simulink model "HDVSim_Hybrid_Truck"  (R2019b)
settings: Solver=ode3  StartTime=0.0  StopTime=inf  FixedStep=sample_time  MaxStep=0.1  RelTol=1e-2  EnableMultiTasking=on
model workspace (MAT data in the model file):
  Bat.SOC_start = 1
  Bat.Voltage = 800
  Bat.Useable_range = 0.8
  Bat.Charge_cycles = 3000
  Bat.Useable_capacity = 61.538
  Bat.Efficiency_power_electronics = 0.95
  Bat.Type = 2
  Electric_Truck = 0
  FuelCell.P_nom = 0
  Fueltype = 4
  Hybrid_Truck = 1
  IZ12 = 3600
  M_el = 500
  SOC_T_EM_completely_available = 0.5
  SOC_addition = 0.4
  SOC_el = 0.8
  SOC_target = 0.85
  Sailing_without_drag_torque = 0
  TCO = TCO
  TCO.Ratio_Trailer_Truck = [1 1]
  TCO.Price_increase_repurchase = [3.5 3.5]
  TCO.bDiesel = 20.3670861225
  TCO.CNG_consumption = 0
  TCO.LNG_consumption = 0
  TCO.energyTotal = 12.8064463441
  TCO.Hydrogen_consumption = 0
  TCO.Daily_working_time = 8
  TCO.Other_works_day = 0.5
  TCO.Gross_hourly_wage_driver = 13
  TCO.Operating_days_year = 240
  TCO.Annual_gross_wage_driver = 24960
  TCO.Personnel_factor = 1.3
  TCO.Annual_gross_wage_truck = 32448
  TCO.Social_costs_percent = 25.75
  TCO.Social_costs_EUR = 8355
  TCO.Back_up_driver = 0
  TCO.Fees_day = 12
  TCO.Fees_year = 2880
  TCO.Driver_costs_other = 0
  TCO.Driving_personnel_costs_total = 43683
  TCO.Diesel_price = 0.95
  TCO.Diesel_price_external = 1
  TCO.Adblue_price = 0.68
  TCO.CNG_price = 0.88
  TCO.LNG_price = 0.81
  TCO.Electricity_price = 0.12
  TCO.Hydrogen_price = 9
  TCO.Interest_current_asset = 8.4
  TCO.Interest_fixed_asset = 8.4
  TCO.Administrative_costs = 10.7
  TCO.Driving_distance = 0
  TCO.Ratio_toll_route = 75
  TCO.Loading_time_combination = 0.25
  TCO.Unloading_time_combination = 0.25
  TCO.Ratio_empty_trips = 0
  TCO.Operating_life = [6 12]
  TCO.Tire_mileage = [140000 170000]
  TCO.Ratio_inhouse_tanking_diesel = 75
  TCO.Lubricant_consumption = 1
  TCO.Adblue_consumption = 4
  TCO.Afa_time_dependent = [100 100]
  TCO.Afa_power_dependent = [0 0]
  TCO.Current_asset = [6000 1400]
  TCO.Insurance_vehicle = [0 0]
  TCO.Insurance_transport = 1000
  TCO.Communication = 600
  TCO.Repair_maintenance_care = [0.13 0.025]
  TCO.Current_assets_interest_bearing = 622
  TCO.Results = table
  TCO.Results.data = {{[]; []; []; []; []; []; []; []; []; []; []; []; []; []; []; []; []; []; []; []; []; []; []}, {[]; []; []; []; []; []; []; []; []; []; []; []; []; []; []; []; []; []; []; []; []; []; []}, {[]; []; []; []; []; []; []; []; []; []; []; []; []; []; []; []; []; []; []; []; []; []; []}, {'#cccccc'; '#9DFF00'; '#CEFF00'; '#FFFF00'; '#FFDF00'; '#FFB900'; '#FF9200'; '#FF5D00'; '#FF7F7F'; '#FF2900'; '#cccccc'; '#00FF85'; '#00FFC6'; '#00FFF2'; '#00FFFF'; '#00DBFF'; '#00B3FF'; '#0088FF'; '#cccccc'; '#B100FF'; '#FF00FF'; '#FF00C0'; '#FF0070'}, [0; 1; 1; 1; 1; 1; 1; 0; 1; 0; 0; 1; 1; 1; 1; 1; 1; 0; 0; 0; 1; 1; 0]}
  TCO.Results.ndims = 2
  TCO.Results.nrows = 23
  TCO.Results.rownames = {'Time_dependent_vehicle_costs'; 'Amortisation_time_dependent'; 'Current_assets_interest_bearing'; 'Fixed_assets_interest_bearing'; 'Taxes'; 'Insurance'; 'Communication'; 'Sum_vehicle_costs'; 'Driving_personnel_costs_total'; 'Sum_fix_costs'; 'Performance_related_vehicle_costs'; 'Amortisation_performance_related'; 'Fuel_costs'; 'Adblue_costs'; 'Repair_costs'; 'Lubricant_costs'; 'Tire_costs'; 'Sum_variable_costs'; 'Sum_costs'; 'Sum_fix_variable_costs'; 'Overhead_costs'; 'Toll_costs'; 'Total_costs'}
  TCO.Results.nvars = 5
  TCO.Results.varnames = {'Euro_Year', 'Euro_km', 'Percent', 'Cellcolor', 'Diagramm'}
  TCO.Results.props.useVariableNamesOriginal = 0
  TCO.Results.props.useDimensionNamesOriginal = 0
  TCO.Results.props.CustomProps = struct()
  TCO.Results.props.VariableCustomProps = struct()
  TCO.Results.props.versionSavedFrom = 4
  TCO.Results.props.minCompatibleVersion = 1
  TCO.Results.props.incompatibilityMsg = ''
  TCO.Results.props.VersionSavedFrom = 4
  TCO.Results.props.Description = ''
  TCO.Results.props.VariableNamesOriginal = {}
  TCO.Results.props.DimensionNames = {'Row', 'Variables'}
  TCO.Results.props.DimensionNamesOriginal = {}
  TCO.Results.props.UserData = []
  TCO.Results.props.VariableDescriptions = {}
  TCO.Results.props.VariableUnits = {}
  TCO.Results.props.VariableContinuity = {}
  TCO_Trailer = 1
  T_distance_LPS_down = 250
  T_distance_LPS_up = 250
  VSim.Display = 3
  VSim.Opt = 0
  VSim.Termination = 1
  Vehicle = 'empty'
  WPT.Voltage = 0
  WPT.P_max = 120
  WPT.expansion = 0.42
  WPT.eta = 0.92
  WPT.SOC_target = 1
  WPT.SOC_electric_only = 1
  acquisitionCosts = acquisitionCosts
  acquisitionCosts.KA_ZM = 128696.075491
  acquisitionCosts.KG = 6766.52227329
  acquisitionCosts.Getr_m = 306.461675078
  acquisitionCosts.KMot = 12284.8
  acquisitionCosts.Motor_Mmax = 2400
  acquisitionCosts.Motor_m = 1122.29
  acquisitionCosts.KH = 20987.6378271
  acquisitionCosts.Hybrid = 1
  acquisitionCosts.Bat = 15579.57696
  acquisitionCosts.Bat_type = 2
  acquisitionCosts.Bat_m = 382.223602484
  acquisitionCosts.Bat_kWh = 61.538
  acquisitionCosts.Bat_VK_Pouch = 176
  acquisitionCosts.Bat_VK_Cyl = 210
  acquisitionCosts.Ratio_BEV2PHEV = 1.42
  acquisitionCosts.EM = 3501.40973218
  acquisitionCosts.EM_Type = 1
  acquisitionCosts.EM_P_max = 272.271363311
  acquisitionCosts.LE = 1225.2211349
  acquisitionCosts.KWPT = 10000
  acquisitionCosts.FC = 0
  acquisitionCosts.P_FC = 0
  acquisitionCosts.KTFC_VK = 608
  acquisitionCosts.KA = 6181
  acquisitionCosts.v_diesel = 500
  acquisitionCosts.v_cng = 0
  acquisitionCosts.v_lng = 0
  acquisitionCosts.m_h2 = 0
  acquisitionCosts.KT = 403.75
  acquisitionCosts.KTD = 403.75
  acquisitionCosts.KTCNG = 0
  acquisitionCosts.KTLNG = 0
  acquisitionCosts.KTFC = 0
  addition_for_critical_slope = 0.01
  ambient.gravity = 9.80665
  ambient.air_density = 1.1883
  ambient.friction_coeff = 0.8
  auxiliary_consumers.onBattery = 0
  auxiliary_consumers.power = 3500
  critical_altitude_difference = 50
  cycle.distance = [0 1 2 3 4 5 6 7 8 9 10 11 ... (92937 elements, 92937x1)]
  cycle.speed = [0 23.0555555556 23.0555555556 23.0555555556 23.0555555556 23.0555555556 23.0555555556 23.0555555556 23.0555555556 23.0555555556 23.0555555556 23.0555555556 ... (92937 elements, 92937x1)]
  cycle.slope = [-0.008925 -0.008925 -0.0089836957 -0.0090130435 -0.0090423913 -0.0090717391 -0.009101087 -0.0091304348 -0.0091597826 -0.0091891304 -0.0092184783 -0.0092478261 ... (92937 elements, 92937x1)]
  cycle.stop_time = [1 1 1 0 0 0 0 0 0 0 0 0 ... (92937 elements, 92937x1)]
  cycle.altitude = [0 0 0 0 0 0 0 0 0 0 0 0 ... (92937 elements, 92937x1)]
  cycle.stop_end = 15
  cycle.stop_start = 5
  cycle.speed_init = 1
  cycle.delta12 = [0 0 0 0 0 0 0 0 0 0 0 0 ... (92937 elements, 1x92937)]
  cycle.delta23 = [0 0 0 0 0 0 0 0 0 0 0 0 ... (92937 elements, 1x92937)]
  dcycle = 21
  distance_1_PPC = 175
  distance_2_PPC = 200
  distance_PPC_altitude_difference = 10000
  driver.controller_proportional_gain = 230000
  driver.controller_integral_gain = 3000
  driver.slew_rate_throttle_in = 2
  driver.slew_rate_throttle_out = -2
  driver.slew_rate_brake_in = 2
  driver.slew_rate_brake_out = -2
  em.P_max = 272.271363311
  em.M_max = 2000
  em.n_eck = 1300
  em.n_max = 2000
  em.shift_parameter.n_pref = 1222
  em.shift_parameter.n2 = 273
  em.shift_parameter.n6 = 1600
  em.shift_parameter.M_max = 2000
  em.fuel.co2_per_kwh = 504
  em.speed = [0 10 20 30 40 50 60 70 80 90 100 110 120 130 140 150 160 170 180 190 200 210 220 230 240 250 260 270 280 290 300 310 320 330 340 350 360 370 380 390 400 410 420 430 440 450 460 470 480 490 500 510 520 530 540 550 560 570 580 590 600 610 620 630 640 650 660 670 680 690 700 710 720 730 740 750 760 770 780 790 800 810 820 830 840 850 860 870 880 890 900 910 920 930 940 950 960 970 980 990 1000 1010 1020 1030 1040 1050 1060 1070 1080 1090 1100 1110 1120 1130 1140 1150 1160 1170 1180 1190 1200 1210 1220 1230 1240 1250 1260 1270 1280 1290 1300 1310 1320 1330 1340 1350 1360 1370 1380 1390 1400 1410 1420 1430 1440 1450 1460 1470 1480 1490 1500 1510 1520 1530 1540 1550 1560 1570 1580 1590 1600 1610 1620 1630 1640 1650 1660 1670 1680 1690 1700 1710 1720 1730 1740 1750 1760 1770 1780 1790 1800 1810 1820 1830 1840 1850 1860 1870 1880 1890 1900 1910 1920 1930 1940 1950 1960 1970 1980 1990 2000]
  em.trq = [2000 2000 2000 2000 2000 2000 2000 2000 2000 2000 2000 2000 2000 2000 2000 2000 2000 2000 2000 2000 2000 2000 2000 2000 2000 2000 2000 2000 2000 2000 2000 2000 2000 2000 2000 2000 2000 2000 2000 2000 2000 2000 2000 2000 2000 2000 2000 2000 2000 2000 2000 2000 2000 2000 2000 2000 2000 2000 2000 2000 2000 2000 2000 2000 2000 2000 2000 2000 2000 2000 2000 2000 2000 2000 2000 2000 2000 2000 2000 2000 2000 2000 2000 2000 2000 2000 2000 2000 2000 2000 2000 2000 2000 2000 2000 2000 2000 2000 2000 2000 2000 2000 2000 2000 2000 2000 2000 2000 2000 2000 2000 2000 2000 2000 2000 2000 2000 2000 2000 2000 2000 2000 2000 2000 2000 2000 2000 2000 2000 2000 2000 1984.73282443 1969.6969697 1954.88721805 1940.29850746 1925.92592593 1911.76470588 1897.81021898 1884.05797101 1870.50359712 1857.14285714 1843.97163121 1830.98591549 1818.18181818 1805.55555556 1793.10344828 1780.82191781 1768.70748299 1756.75675676 1744.96644295 1733.33333333 1721.85430464 1710.52631579 1699.34640523 1688.31168831 1677.41935484 1666.66666667 1656.05095541 1645.56962025 1635.22012579 1625 1614.9068323 1604.9382716 1595.09202454 1585.36585366 1575.75757576 1566.26506024 1556.88622754 1547.61904762 1538.46153846 1529.41176471 1520.46783626 1511.62790698 1502.89017341 1494.25287356 1485.71428571 1477.27272727 1468.92655367 1460.6741573 1452.51396648 1444.44444444 1436.4640884 1428.57142857 1420.76502732 1413.04347826 1405.40540541 1397.84946237 1390.37433155 1382.9787234 1375.66137566 1368.42105263 1361.2565445 1354.16666667 1347.15025907 1340.20618557 1333.33333333 1326.53061224 1319.79695431 1313.13131313 1306.53266332 1300]
  em.Type = 1
  em.efficiency.characteristic_map = [0.001 0.001 0.001 0.001 0.001 0.001 0.001 0.001 0.001 0.001 0.001 0.001 ... (20301 elements, 101x201)]
  em.efficiency.speed = [0 10 20 30 40 50 60 70 80 90 100 110 120 130 140 150 160 170 180 190 200 210 220 230 240 250 260 270 280 290 300 310 320 330 340 350 360 370 380 390 400 410 420 430 440 450 460 470 480 490 500 510 520 530 540 550 560 570 580 590 600 610 620 630 640 650 660 670 680 690 700 710 720 730 740 750 760 770 780 790 800 810 820 830 840 850 860 870 880 890 900 910 920 930 940 950 960 970 980 990 1000 1010 1020 1030 1040 1050 1060 1070 1080 1090 1100 1110 1120 1130 1140 1150 1160 1170 1180 1190 1200 1210 1220 1230 1240 1250 1260 1270 1280 1290 1300 1310 1320 1330 1340 1350 1360 1370 1380 1390 1400 1410 1420 1430 1440 1450 1460 1470 1480 1490 1500 1510 1520 1530 1540 1550 1560 1570 1580 1590 1600 1610 1620 1630 1640 1650 1660 1670 1680 1690 1700 1710 1720 1730 1740 1750 1760 1770 1780 1790 1800 1810 1820 1830 1840 1850 1860 1870 1880 1890 1900 1910 1920 1930 1940 1950 1960 1970 1980 1990 2000]
  em.efficiency.torque = [0 20 40 60 80 100 120 140 160 180 200 220 240 260 280 300 320 340 360 380 400 420 440 460 480 500 520 540 560 580 600 620 640 660 680 700 720 740 760 780 800 820 840 860 880 900 920 940 960 980 1000 1020 1040 1060 1080 1100 1120 1140 1160 1180 1200 1220 1240 1260 1280 1300 1320 1340 1360 1380 1400 1420 1440 1460 1480 1500 1520 1540 1560 1580 1600 1620 1640 1660 1680 1700 1720 1740 1760 1780 1800 1820 1840 1860 1880 1900 1920 1940 1960 1980 2000]
  em.noEM = 2
  em_M_max = 678.4739048
  engine.bsfc.be = [1463.81124945 1337.51367643 1219.93532707 1127.30261663 1034.66990619 942.037195751 849.40448531 756.771774869 664.139064428 571.506353987 478.873643547 386.240933106 ... (45451 elements, 301x151)]
  engine.bsfc.trqorig = [0 10 20 30 40 50 60 70 80 90 100 110 120 130 140 150 160 170 180 190 200 210 220 230 240 250 260 270 280 290 300 310 320 330 340 350 360 370 380 390 400 410 420 430 440 450 460 470 480 490 500 510 520 530 540 550 560 570 580 590 600 610 620 630 640 650 660 670 680 690 700 710 720 730 740 750 760 770 780 790 800 810 820 830 840 850 860 870 880 890 900 910 920 930 940 950 960 970 980 990 1000 1010 1020 1030 1040 1050 1060 1070 1080 1090 1100 1110 1120 1130 1140 1150 1160 1170 1180 1190 1200 1210 1220 1230 1240 1250 1260 1270 1280 1290 1300 1310 1320 1330 1340 1350 1360 1370 1380 1390 1400 1410 1420 1430 1440 1450 1460 1470 1480 1490 1500 1510 1520 1530 1540 1550 1560 1570 1580 1590 1600 1610 1620 1630 1640 1650 1660 1670 1680 1690 1700 1710 1720 1730 1740 1750 1760 1770 1780 1790 1800 1810 1820 1830 1840 1850 1860 1870 1880 1890 1900 1910 1920 1930 1940 1950 1960 1970 1980 1990 2000 2010 2020 2030 2040 2050 2060 2070 2080 2090 2100 2110 2120 2130 2140 2150 2160 2170 2180 2190 2200 2210 2220 2230 2240 2250 2260 2270 2280 2290 2300 2310 2320 2330 2340 2350 2360 2370 2380 2390 2400 2410 2420 2430 2440 2450 2460 2470 2480 2490 2500 2510 2520 2530 2540 2550 2560 2570 2580 2590 2600 2610 2620 2630 2640 2650 2660 2670 2680 2690 2700 2710 2720 2730 2740 2750 2760 2770 2780 2790 2800 2810 2820 2830 2840 2850 2860 2870 2880 2890 2900 2910 2920 2930 2940 2950 2960 2970 2980 2990 3000]
  engine.bsfc.speed = [600 610 620 630 640 650 660 670 680 690 700 710 720 730 740 750 760 770 780 790 800 810 820 830 840 850 860 870 880 890 900 910 920 930 940 950 960 970 980 990 1000 1010 1020 1030 1040 1050 1060 1070 1080 1090 1100 1110 1120 1130 1140 1150 1160 1170 1180 1190 1200 1210 1220 1230 1240 1250 1260 1270 1280 1290 1300 1310 1320 1330 1340 1350 1360 1370 1380 1390 1400 1410 1420 1430 1440 1450 1460 1470 1480 1490 1500 1510 1520 1530 1540 1550 1560 1570 1580 1590 1600 1610 1620 1630 1640 1650 1660 1670 1680 1690 1700 1710 1720 1730 1740 1750 1760 1770 1780 1790 1800 1810 1820 1830 1840 1850 1860 1870 1880 1890 1900 1910 1920 1930 1940 1950 1960 1970 1980 1990 2000 2010 2020 2030 2040 2050 2060 2070 2080 2090 2100]
  engine.bsfc.M_be_min_orig = [1000 1040 1080 1120 1130 1140 1150 1160 1170 1180 1190 1200 1480 1520 1560 1600 1640 1680 1720 1760 1800 1840 1880 1920 1960 2000 2040 2080 2120 2160 2200 2220 2240 2240 2240 2240 2230 2230 2140 2130 2130 2130 2130 2130 2130 2130 2130 2030 1840 1840 1830 1830 1830 1830 1830 1830 1830 1830 1830 1730 1730 1730 1730 1730 1720 1720 1720 1720 1720 1720 1720 1720 1720 1720 1720 1720 1720 1720 1720 1720 1710 1720 1710 1710 1710 1620 1620 1620 1620 1620 1620 1630 1640 1640 1650 1660 1670 1680 1690 1700 1710 1710 1710 1710 1710 1710 1710 1710 1710 1710 1900 1890 1890 1890 1890 1890 1890 1890 1880 1870 1860 1840 1830 1820 1810 1800 1780 1770 1760 1750 1740 1720 1710 1700 1690 1680 1660 1650 1640 1630 1620 0 0 0 0 0 0 0 0 0 0]
  engine.bsfc.trq = [0 10 20 30 40 50 60 70 80 90 100 110 120 130 140 150 160 170 180 190 200 210 220 230 240 250 260 270 280 290 300 310 320 330 340 350 360 370 380 390 400 410 420 430 440 450 460 470 480 490 500 510 520 530 540 550 560 570 580 590 600 610 620 630 640 650 660 670 680 690 700 710 720 730 740 750 760 770 780 790 800 810 820 830 840 850 860 870 880 890 900 910 920 930 940 950 960 970 980 990 1000 1010 1020 1030 1040 1050 1060 1070 1080 1090 1100 1110 1120 1130 1140 1150 1160 1170 1180 1190 1200 1210 1220 1230 1240 1250 1260 1270 1280 1290 1300 1310 1320 1330 1340 1350 1360 1370 1380 1390 1400 1410 1420 1430 1440 1450 1460 1470 1480 1490 1500 1510 1520 1530 1540 1550 1560 1570 1580 1590 1600 1610 1620 1630 1640 1650 1660 1670 1680 1690 1700 1710 1720 1730 1740 1750 1760 1770 1780 1790 1800 1810 1820 1830 1840 1850 1860 1870 1880 1890 1900 1910 1920 1930 1940 1950 1960 1970 1980 1990 2000 2010 2020 2030 2040 2050 2060 2070 2080 2090 2100 2110 2120 2130 2140 2150 2160 2170 2180 2190 2200 2210 2220 2230 2240 2250 2260 2270 2280 2290 2300 2310 2320 2330 2340 2350 2360 2370 2380 2390 2400 2410 2420 2430 2440 2450 2460 2470 2480 2490 2500 2510 2520 2530 2540 2550 2560 2570 2580 2590 2600 2610 2620 2630 2640 2650 2660 2670 2680 2690 2700 2710 2720 2730 2740 2750 2760 2770 2780 2790 2800 2810 2820 2830 2840 2850 2860 2870 2880 2890 2900 2910 2920 2930 2940 2950 2960 2970 2980 2990 3000]
  engine.bsfc.M_be_min = [1000 1040 1080 1120 1130 1140 1150 1160 1170 1180 1190 1200 1480 1520 1560 1600 1640 1680 1720 1760 1800 1840 1880 1920 1960 2000 2040 2080 2120 2160 2200 2220 2240 2240 2240 2240 2230 2230 2140 2130 2130 2130 2130 2130 2130 2130 2130 2030 1840 1840 1830 1830 1830 1830 1830 1830 1830 1830 1830 1730 1730 1730 1730 1730 1720 1720 1720 1720 1720 1720 1720 1720 1720 1720 1720 1720 1720 1720 1720 1720 1710 1720 1710 1710 1710 1620 1620 1620 1620 1620 1620 1630 1640 1640 1650 1660 1670 1680 1690 1700 1710 1710 1710 1710 1710 1710 1710 1710 1710 1710 1900 1890 1890 1890 1890 1890 1890 1890 1880 1870 1860 1840 1830 1820 1810 1800 1780 1770 1760 1750 1740 1720 1710 1700 1690 1680 1660 1650 1640 1630 1620 0 0 0 0 0 0 0 0 0 0]
  engine.full_load.speed = [600; 900; 1000; 1350; 2000; 2001; 2100]
  engine.full_load.trqorig = [1000; 2200; 2400; 2400; 1620; 0; 0]
  engine.full_load.trq = [1000; 2200; 2400; 2400; 1620; 0; 0]
  engine.full_load.power = [62.8318530718; 207.345115137; 251.327412287; 339.292006588; 339.292006588; 0; 0]
  engine.number = 1
  engine.M_max_original = 2400
  engine.M_max = 2400
  engine.scale_factor = 1
  engine.fuel.density = 830
  engine.fuel.co2_per_litre = 2.62
  engine.fuel.heat_of_combustion = 42500
  engine.fuel.LHV = 35.8
  engine.speed_min = 600
  engine.speed_max = 2100
  engine.idle_fuel_consumption = 1.5
  engine.drag_torque.trq = [0 -65.6 -98.9 -123.3 -134.32 -156.04 -176.72 -217.28 -234.63 -256.02 -273.98 -301.74 -324 -353.92 -376.32 -421.26 -450]
  engine.drag_torque.speed = [0 450 600 700 800 1000 1200 1500 1600 1700 1800 1900 2000 2100 2200 2300 2400]
  engine.rated_power = 323619.35891
  engine.shift_parameter.n_lo = 1000
  engine.shift_parameter.n_pref = 1200
  engine.shift_parameter.n1 = 600
  engine.shift_parameter.n2 = 800
  engine.shift_parameter.n3 = 1100
  engine.shift_parameter.n4 = 600
  engine.shift_parameter.n5 = 1200
  engine.shift_parameter.n6 = 2100
  engine.shift_parameter.M_max = 2400
  final_drive.ratio = 10
  final_drive.trq_eff = 0.98
  ifElectric = 1
  ifOptimized = 0
  look_ahead_PPC = 250
  max_distance = 100185
  name = 'HDVSim_Hybrid_Truck'
  preditiveCruiseControl = 0
  prospective_cruise_control = 1
  sample_time = 0.1
  tank.v_diesel = 500
  tank.v_cng = 0
  tank.v_lng = 0
  tank.m_h2 = 0
  tires.radius = 0.4465
  tires.roll_drag_coeff.total = 0.0043
  transmission.init_gear = 1
  transmission.shift_time = 1
  transmission.ratios = [15.86 12.3362940231 9.59546974938 7.4635899192 5.80536189857 4.51555178382 3.51230608335 2.73195715911 2.12498277259 1.65286332136 1.28563732108 1]
  transmission.trq_eff = [0.96 0.96 0.96 0.96 0.96 0.98 0.97 0.97 0.97 0.97 0.97 0.99]
  transmission.lamda = [19.5082940255 8.7020449746 4.12999864633 2.25518525425 1.51407033279 1.23026605467 1.12010939473 1.07005906552 1.04003695 1.0240124152 1.01900932897 1.0130058518]
  transmission.z = 12
  v_PPC_delta = 2.77777777778
  v_max_electrical_drive_only = 13.8888888889
  vehicle.long_truck = 0
  vehicle.payload = 19300
  vehicle.frontal_area = 10.2
  vehicle.air_drag_coeff = 0.53
  vehicle.Fx_max = 90221.18
  vehicle.F_brake_max = 309890.14
  vehicle.mass = 13372.577731
  vehicleProperties = vehicleProperties
  vehicleProperties.CO2_EM_Range = 100
  vehicleProperties.a_0_80_Range = 54
  vehicleProperties.BOK_ak = 2.55
  vehicleProperties.BOK_Range = 4.65
  vehicleProperties.V_Rel_ak = 0.55
  vehicleProperties.V_Rel_Range = 0.35
  vehicleProperties.VWF_ak = 0.9
  vehicleProperties.VWF_Range = 7.2
  vehicle_F_brake_max = 309890.14
  weights = weights
  weights.m_Engine = 1122.29
  weights.m_Gearbox = 306.461675078
  weights.m_Retarder = 82
  weights.m_tank_system = 51.6564603608
  weights.m_Fuel = 425
  weights.m_Exhaust = 203.4
  weights.m_Battery = 382.223602484
  weights.m_EM = 136.135681656
  weights.m_PwrElectr = 25.2103114177
  weights.m_Charger = 15
  weights.m_Trailer = 5400
  weights.m_Total = 7972.577731
  weights.m_Base = 5223.2
  weights.Hybrid = 1
  weights.max_Payload = 27627.422269
  weights.m_Max = 41000
  x = 1
values from neighbouring files (symbols this model uses):
  transmission = Transmission_gearing(15.86, 7, 0, 0, final_drive.ratio)    [Parameterizing.m]
undefined symbols (not defined in the file or in its folder): Hybrid_LKW, Ion, Lithium
libraries: simulink x23, powerlib x2, electricdrivelib x1
(part 1 of 3)
settings Model: NumberOfSteps=500  Creator=Alexander Suessmann  LastModifiedBy=ubuntu
settings DataIOCC: SaveFormat=Array  SaveOutput=off  SignalLogging=off  DSMLogging=off  ReturnWorkspaceOutputs=off  SignalLoggingName=datalog
settings OptimizationCC: DefaultParameterBehavior=Inlined  ExpressionDepthLimit=2147483647  InitFltsAndDblsToZero=on  LifeSpan=inf
settings DebuggingCC: UnderspecifiedInitializationDetection=Classic  MergeDetectMultiDrivingBlocksExec=none  SolverPrmCheckMsg=warning  MultiTaskDSMMsg=warning  MultiTaskCondExecSysMsg=none  FcnCallInpInsideContextMsg=warning  AllowSymbolicDim=off  StateNameClashWarn=warning  SFUnusedDataAndEventsDiag=none  SFUnexpectedBacktrackingDiag=none  SFInvalidInputDataAccessInChartInitDiag=none  SFNoUnconditionalDefaultTransitionDiag=none  SFTransitionOutsideNaturalParentDiag=none  SFUnreachableExecutionPathDiag=none  SFUndirectedBroadcastEventsDiag=none  SFTransitionActionBeforeConditionDiag=none  SFExecutionAtInitializationDiag=none  ArithmeticOperatorsInVariantConditions=warning
settings HardwareCC: ProdBitPerPointer=32  ProdBitPerSizeT=32  ProdBitPerPtrDiffT=32  ProdLargestAtomicFloat=None  ProdIntDivRoundTo=Undefined  ProdEndianess=Unspecified  ProdWordSize=32  ProdHWDeviceType=32-bit Generic
settings ModelReferenceCC: PropagateSignalLabelsOutOfModel=off
settings SFSimCC: SimUseLocalCustomCode=off  CustomCodeUndefinedFunction=UseInterfaceOnly
settings RTWCC: RTWUseLocalCustomCode=off
settings CodeAppCC: StateflowObjectComments=on
settings GRTTargetCC: TargetLangStandard=C89/C90 (ANSI)  GeneratePreprocessorConditionals=Disable all  CombineOutputUpdateFcns=off  GRTInterface=on
settings ConfigComp: CovMetricSettings=dw  CovHtmlReporting=on  CovSaveCumulativeToWorkspaceVar=on  CovSaveSingleToWorkspaceVar=on  CovExternalEMLEnable=off  CovSFcnEnable=off
settings SimscapeCC: SimscapeNormalizeSystem=off

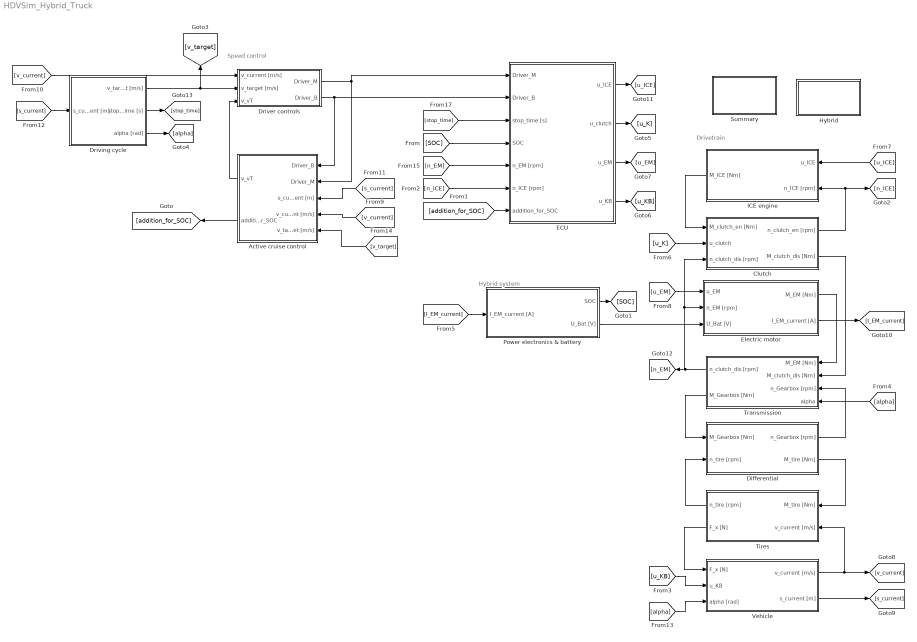
== system HDVSim_Hybrid_Truck ==
  note: Hybrid system
  note: Speed control
  note: Drivetrain
  Active cruise control : Subsystem
  Clutch : Subsystem
  Differential : Subsystem
  Driver controls : Subsystem
  Driving cycle : Subsystem
  ECU : Subsystem
  Electric motor : Subsystem
  Hybrid : Subsystem
  ICE engine : Subsystem
  Power electronics & battery : Subsystem
  Summary : Subsystem
  Tires : Subsystem
  Transmission : Subsystem
  Vehicle : Subsystem
  wires:
  ICE engine/1(M_ICE [Nm]) -> Clutch/1(M_clutch_en [Nm])
  Transmission/1(n_clutch_dis [rpm]) -> Clutch/3(n_clutch_dis [rpm]), Electric motor/2(n_EM [rpm]), [n_EM]
  Transmission/2(M_Gearbox [Nm]) -> Differential/1(M_Gearbox [Nm])
  Tires/1(n_tire [rpm]) -> Differential/2(n_tire [rpm])
  Clutch/1(n_clutch_en [rpm]) -> [n_ICE], ICE engine/2(n_ICE [rpm])
  Clutch/2(M_clutch_dis [Nm]) -> Transmission/2(M_clutch_dis [Nm])
  Differential/1(n_Gearbox [rpm]) -> Transmission/3(n_Gearbox [rpm])
  Differential/2(M_tire [Nm]) -> Tires/1(M_tire [Nm])
  Vehicle/1(v_current [m/s]) -> [v_current], Tires/2(v_current [m/s])
  Tires/2(F_x [N]) -> Vehicle/1(F_x [N])
  Driver controls/2(Driver_B) -> Active cruise control/1(Driver_B), ECU/2(Driver_B)
  Electric motor/1(M_EM [Nm]) -> Transmission/1(M_EM [Nm])
  Active cruise control/2(addition_for_SOC) -> [addition_for_SOC]
  [addition_for_SOC] -> ECU/7(addition_for_SOC)
  Power electronics & battery/1(SOC) -> [SOC]
  [SOC] -> ECU/4(SOC)
  [n_ICE] -> ECU/6(n_ICE [rpm])
  Driving cycle/1(v_target [m/s]) -> [v_target], Driver controls/2(v_target [m/s])
  [v_current] -> Driver controls/1(v_current [m/s])
  [s_current] -> Driving cycle/1(s_current [m])
  Driving cycle/3(alpha [rad]) -> [alpha]
  Active cruise control/1(v_vT) -> Driver controls/3(v_vT)
  Driver controls/1(Driver_M) -> Active cruise control/2(Driver_M), ECU/1(Driver_M)
  [s_current] -> Active cruise control/3(s_current [m])
  [v_current] -> Active cruise control/4(v_current [m/s])
  [v_target] -> Active cruise control/5(v_target [m/s])
  Power electronics & battery/2(U_Bat [V]) -> Electric motor/3(U_Bat [V])
  [u_EM] -> Electric motor/1(u_EM)
  ECU/2(u_clutch) -> [u_K]
  [u_K] -> Clutch/2(u_clutch)
  ECU/4(u_KB) -> [u_KB]
  [u_KB] -> Vehicle/2(u_KB)
  [alpha] -> Vehicle/3(alpha [rad])
  Vehicle/2(s_current [m]) -> [s_current]
  [alpha] -> Transmission/4(alpha)
  Electric motor/2(I_EM_current [A]) -> [I_EM_current]
  [I_EM_current] -> Power electronics & battery/1(I_EM_current [A])
  ECU/3(u_EM) -> [u_EM]
  ECU/1(u_ICE) -> [u_ICE]
  [u_ICE] -> ICE engine/1(u_ICE)
  [n_EM] -> ECU/5(n_EM [rpm])
  Driving cycle/2(stop_time [s]) -> [stop_time]
  [stop_time] -> ECU/3(stop_time [s])

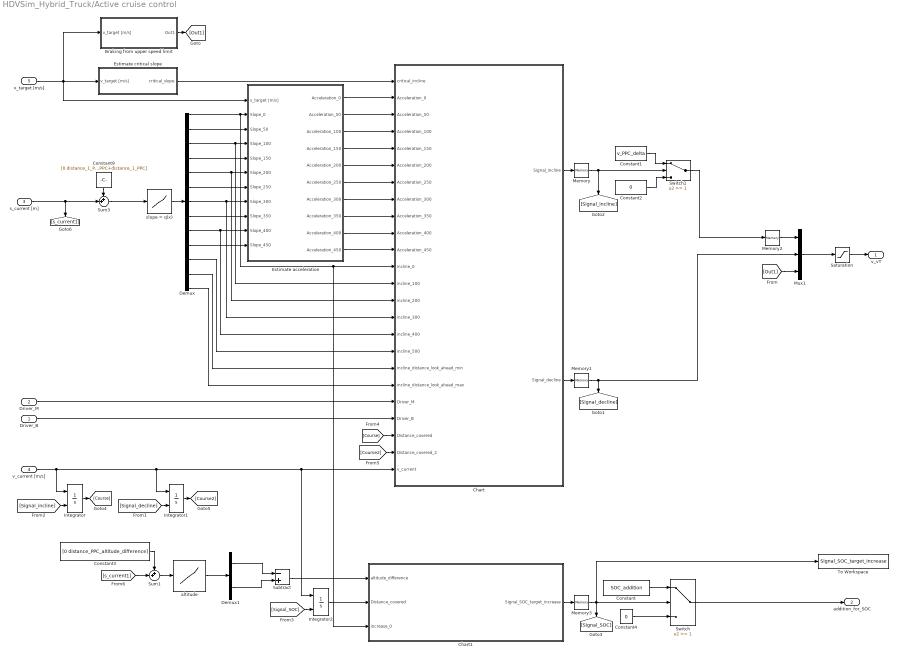
== system HDVSim_Hybrid_Truck/Active cruise control ==
  Driver_B : Inport
  Driver_M : Inport  Port=2
  s_current [m] : Inport  Port=3
  v_current [m/s] : Inport  Port=4
  v_target [m/s] : Inport  Port=5
  Braking from upper speed limit : Subsystem
  Chart : Stateflow chart  TreatAsAtomicUnit=on
  Chart1 : Stateflow chart  TreatAsAtomicUnit=on
  Constant : Constant  Value=SOC_addition
  Constant1 : Constant  Value=v_PPC_delta
  Constant2 : Constant  Value=0
  Constant3 : Constant  Value=[0 distance_PPC_altitude_difference]
  Constant4 : Constant  Value=0
  Constant9 : Constant  Value=[0 distance_1_PPC/5 2*distance_1_PPC/5 3*distance_1_PPC/5 4*distance_1_PPC/5 distance_1_PPC 6*distance_1_PPC/5 7*distance_1_PPC/5 8*distance_1_PPC/5 9*distance_1_PPC/5 10*distance_1_PPC/5 look_ahead_PPC look_ahead_PPC+distance_1_PPC]
  Demux : Demux  Outputs=13
  Demux1 : Demux  Outputs=2
  Estimate acceleration : Subsystem
  Estimate critical slope : Subsystem
  Integrator : Integrator  ExternalReset=rising
  Integrator1 : Integrator  ExternalReset=rising
  Integrator2 : Integrator  ExternalReset=rising
  Memory{,1..3} (4 alike) : Memory
  Mux1 : Mux  Inputs=3
  Saturation : Saturate  UpperLimit=92/3.6  LowerLimit=0
  Subtract : Sum  Inputs=-+
  Sum1 : Sum  Inputs=++|
  Sum3 : Sum  Inputs=++|
  Switch : Switch  Threshold=1
  Switch1 : Switch  Threshold=1
  To Workspace : ToWorkspace  VariableName=Signal_SOC_target_increase  SaveFormat=Structure With Time  SampleTime=-1
  altitude : Lookup_n-D  NumberOfTableDimensions=1  BreakpointsForDimension1=cycle.distance  Table=cycle.altitude  InterpMethod=Flat  RndMeth=Simplest  InputSameDT=on
  slope = q(x) : Lookup  InputValues=cycle.distance  Table=cycle.slope  LookUpMeth=Interpolation-Use End Values
  v_vT : Outport  VectorParamsAs1DForOutWhenUnconnected=off
  addition_for_SOC : Outport  Port=2  VectorParamsAs1DForOutWhenUnconnected=off
  wires:
  slope = q(x)/1 -> Demux/1
  Demux/1 -> Chart1/3(increase_0), Chart/12(incline_0), Estimate acceleration/2(Slope_0)
  Estimate critical slope/1(critical_slope) -> Chart/1(critical_incline)
  Chart/1(Signal_incline) -> Memory/1
  v_current [m/s]/1 -> Integrator/1, Integrator1/1, Chart/24(v_current), Integrator2/1
  Memory/1 -> [Signal_incline], Switch1/2
  Mux1/1 -> Saturation/1
  Constant9/1 -> Sum3/1
  Sum3/1 -> slope = q(x)/1
  Estimate acceleration/1(Acceleration_0) -> Chart/2(Acceleration_0)
  Estimate acceleration/2(Acceleration_50) -> Chart/3(Acceleration_50)
  Estimate acceleration/3(Acceleration_100) -> Chart/4(Acceleration_100)
  Estimate acceleration/4(Acceleration_150) -> Chart/5(Acceleration_150)
  Estimate acceleration/5(Acceleration_200) -> Chart/6(Acceleration_200)
  Estimate acceleration/6(Acceleration_250) -> Chart/7(Acceleration_250)
  Estimate acceleration/7(Acceleration_300) -> Chart/8(Acceleration_300)
  Estimate acceleration/8(Acceleration_350) -> Chart/9(Acceleration_350)
  Chart/2(Signal_decline) -> Memory1/1
  Memory1/1 -> Mux1/2, [Signal_decline]
  Driver_B/1 -> Chart/21(Driver_B)
  Estimate acceleration/9(Acceleration_400) -> Chart/10(Acceleration_400)
  Estimate acceleration/10(Acceleration_450) -> Chart/11(Acceleration_450)
  Driver_M/1 -> Chart/20(Driver_M)
  Demux/2 -> Estimate acceleration/3(Slope_50)
  Demux/3 -> Chart/13(incline_100), Estimate acceleration/4(Slope_100)
  Demux/4 -> Estimate acceleration/5(Slope_150)
  Demux/5 -> Chart/14(incline_200), Estimate acceleration/6(Slope_200)
  Demux/6 -> Estimate acceleration/7("Slope_250 ")
  Demux/7 -> Chart/15(incline_300), Estimate acceleration/8(Slope_300)
  Demux/8 -> Estimate acceleration/9(Slope_350)
  Demux/9 -> Chart/16(incline_400), Estimate acceleration/10(Slope_400)
  Demux/10 -> Estimate acceleration/11(Slope_450)
  Demux/12 -> Chart/18(incline_distance_look_ahead_min)
  Demux/13 -> Chart/19(incline_distance_look_ahead_max)
  v_target [m/s]/1 -> Braking from upper speed limit/1(v_target [m/s]), Estimate acceleration/1(v_target [m/s]), Estimate critical slope/1(v_target [m/s])
  Memory2/1 -> Mux1/1
  Constant1/1 -> Switch1/1
  Constant2/1 -> Switch1/3
  Demux/11 -> Chart/17(incline_500)
  Switch1/1 -> Memory2/1
  Constant3/1 -> Sum1/1
  s_current [m]/1 -> [s_current1], Sum3/2
  Sum1/1 -> altitude/1
  altitude/1 -> Demux1/1
  Demux1/2 -> Subtract/2
  Demux1/1 -> Subtract/1
  Subtract/1 -> Chart1/1(altitude_difference)
  Chart1/1(Signal_SOC_target_increase) -> Memory3/1
  Integrator2/1 -> Chart1/2(Distance_covered)
  Constant/1 -> Switch/1
  Constant4/1 -> Switch/3
  Switch/1 -> addition_for_SOC/1
  Memory3/1 -> Switch/2, To Workspace/1, [Signal_SOC]
  [Signal_decline] -> Integrator1/2
  Integrator1/1 -> [Course2]
  Integrator/1 -> [Course]
  [Signal_incline] -> Integrator/2
  [Course] -> Chart/22(Distance_covered)
  [Course2] -> Chart/23(Distance_covered_2)
  [Signal_SOC] -> Integrator2/2
  [s_current1] -> Sum1/2
  Braking from upper speed limit/1(Out1) -> [Out1]
  [Out1] -> Mux1/3
  Saturation/1 -> v_vT/1

== system HDVSim_Hybrid_Truck/Clutch ==
  note: Torque where clutch is disengaged
  note: Speed where clutch is engaged
  M_clutch_en [Nm] : Inport
  u_clutch : Inport  Port=2
  n_clutch_dis [rpm] : Inport  Port=3
  Compare To Zero : simulink/Logic and Bit Operations/Compare To Zero  relop===  OutDataTypeStr=boolean
  Constant : Constant  Value=0
  Idle engine speed : Constant  Value=engine.speed_min
  Logical Operator : Logic  AllPortsSameDT=off  OutDataTypeStr=boolean
  Lower engine speed : Saturate  UpperLimit=10000  LowerLimit=engine.speed_min  ZeroCross=off
  Switch1 : Switch  Threshold=1
  Switch2 : Switch  Threshold=1
  Switch3 : Switch  Threshold=1
  zero1 : Constant  Value=0
  n_clutch_en [rpm] : Outport  VectorParamsAs1DForOutWhenUnconnected=off
  M_clutch_dis [Nm] : Outport  Port=2  VectorParamsAs1DForOutWhenUnconnected=off
  wires:
  n_clutch_dis [rpm]/1 -> Lower engine speed/1
  Lower engine speed/1 -> Switch3/3
  Switch2/1 -> Switch1/3
  zero1/1 -> Switch2/1
  M_clutch_en [Nm]/1 -> Switch2/3
  [M_mot] -> Compare To Zero/1
  Compare To Zero/1 -> Logical Operator/1
  [ZKU] -> Logical Operator/2
  Logical Operator/1 -> Switch2/2
  Switch1/1 -> M_clutch_dis [Nm]/1
  Constant/1 -> Switch1/1
  Switch3/1 -> n_clutch_en [rpm]/1
  u_clutch/1 -> Switch1/2, Switch3/2
  Idle engine speed/1 -> Switch3/1

== system HDVSim_Hybrid_Truck/Differential ==
  note: Effective wheel torque
  M_Gearbox [Nm] : Inport
  n_tire [rpm] : Inport  Port=2
  Differential efficiency : Constant  Value=final_drive.trq_eff
  Differential gear ratio : Constant  Value=final_drive.ratio
  M' = M*i*eta : Product  Inputs=***
  Product : Product  Inputs=/  RndMeth=Zero
  Switch : Switch  ZeroCross=off
  n' *i = n : Product  RndMeth=Zero
  n_Gearbox [rpm] : Outport  VectorParamsAs1DForOutWhenUnconnected=off
  M_tire [Nm] : Outport  Port=2  VectorParamsAs1DForOutWhenUnconnected=off
  tag scope: Eta_Axle_drive=global
  wires:
  M_Gearbox [Nm]/1 -> M' = M*i*eta/1, Switch/2
  n_tire [rpm]/1 -> n' *i = n/2
  Differential gear ratio/1 -> n' *i = n/1, M' = M*i*eta/3
  n' *i = n/1 -> n_Gearbox [rpm]/1
  M' = M*i*eta/1 -> M_tire [Nm]/1
  Differential efficiency/1 -> Product/1, Switch/1
  Product/1 -> Switch/3
  Switch/1 -> [Eta_Axle_drive], M' = M*i*eta/2

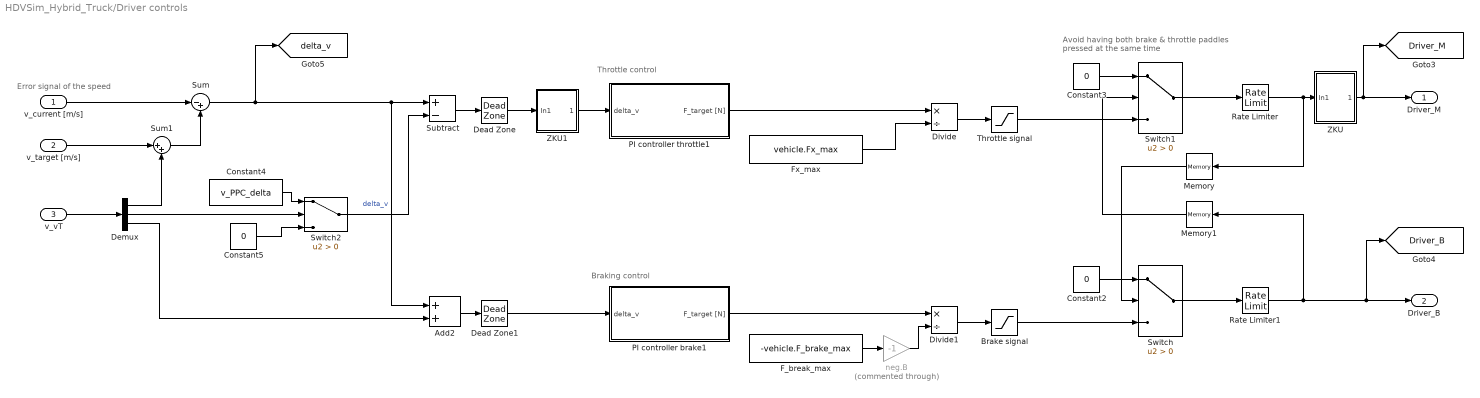
== system HDVSim_Hybrid_Truck/Driver controls ==
  note: Avoid having both brake & throttle
      | paddles pressed at the same time
  note: Throttle control
  note: Braking control
  note: Error signal of the speed
  v_current [m/s] : Inport
  v_target [m/s] : Inport  Port=2
  v_vT : Inport  Port=3
  Add2 : Sum
  Brake signal : Saturate  UpperLimit=1  LowerLimit=0  ZeroCross=off
  Constant2 : Constant  Value=0
  Constant3 : Constant  Value=0
  Constant4 : Constant  Value=v_PPC_delta
  Constant5 : Constant  Value=0
  Dead Zone : DeadZone  LowerValue=-0.1/3.6  UpperValue=0.1/3.6  ZeroCross=off  SaturateOnIntegerOverflow=on
  Dead Zone1 : DeadZone  LowerValue=-0.1/3.6  UpperValue=0  ZeroCross=off  SaturateOnIntegerOverflow=on
  Demux : Demux  Outputs=3
  Divide : Product  Inputs=*/
  Divide1 : Product  Inputs=*/
  F_break_max : Constant  Value=-vehicle.F_brake_max
  Fx_max : Constant  Value=vehicle.Fx_max
  Memory : Memory
  Memory1 : Memory
  PI controller brake1 : Subsystem
  PI controller throttle1 : Subsystem
  Rate Limiter : RateLimiter  RisingSlewLimit=driver.slew_rate_throttle_in  FallingSlewLimit=driver.slew_rate_throttle_out  SampleTimeMode=inherited
  Rate Limiter1 : RateLimiter  RisingSlewLimit=driver.slew_rate_brake_in*100  FallingSlewLimit=driver.slew_rate_brake_out*100  SampleTimeMode=inherited
  Subtract : Sum  Inputs=+-
  Sum : Sum  Inputs=|-+
  Sum1 : Sum  Inputs=|++
  Switch : Switch  Criteria=u2 > Threshold
  Switch1 : Switch  Criteria=u2 > Threshold
  Switch2 : Switch  Criteria=u2 > Threshold
  Throttle signal : Saturate  UpperLimit=1  LowerLimit=0  ZeroCross=off
  ZKU : Subsystem
  ZKU1 : Subsystem
  neg.B : Gain  Commented=through  Gain=-1
  Driver_M : Outport  VectorParamsAs1DForOutWhenUnconnected=off
  Driver_B : Outport  Port=2  VectorParamsAs1DForOutWhenUnconnected=off
  tag scope: Driver_B=global, Driver_M=global, delta_v=global
  wires:
  Sum1/1 -> Sum/2
  v_current [m/s]/1 -> Sum/1
  Fx_max/1 -> Divide/2
  neg.B/1 -> Divide1/2
  Sum/1 -> [delta_v], Add2/1, Subtract/1  "delta_v"
  Divide1/1 -> Brake signal/1
  Divide/1 -> Throttle signal/1
  F_break_max/1 -> neg.B/1
  Switch/1 -> Rate Limiter1/1
  Brake signal/1 -> Switch/3
  Constant2/1 -> Switch/1
  Switch1/1 -> Rate Limiter/1
  Throttle signal/1 -> Switch1/3
  Rate Limiter1/1 -> Memory1/1, [Driver_B], Driver_B/1
  Rate Limiter/1 -> ZKU/1(In1), Memory/1
  Constant3/1 -> Switch1/1
  PI controller throttle1/1(F_target [N]) -> Divide/1
  Dead Zone/1 -> ZKU1/1(In1)
  Dead Zone1/1 -> PI controller brake1/1(delta_v)
  PI controller brake1/1(F_target [N]) -> Divide1/1
  ZKU/1(Out1) -> [Driver_M], Driver_M/1
  ZKU1/1(Out1) -> PI controller throttle1/1(delta_v)
  Memory/1 -> Switch/2
  Memory1/1 -> Switch1/2
  v_vT/1 -> Demux/1
  Switch2/1 -> Subtract/2
  Demux/2 -> Switch2/2
  Constant4/1 -> Switch2/1
  Constant5/1 -> Switch2/3
  Subtract/1 -> Dead Zone/1
  Demux/1 -> Sum1/2
  v_target [m/s]/1 -> Sum1/1
  Demux/3 -> Add2/2
  Add2/1 -> Dead Zone1/1

== system HDVSim_Hybrid_Truck/Driving cycle ==
  note: Lookup tables
  s_current [m] : Inport
  Clock : Clock
  Compare To Constant : simulink/Logic and Bit Operations/Compare To Constant  relop=>=  const=max_distance  OutDataTypeStr=boolean
  Constant : Constant  Value=0
  Holding time at the beginning : Subsystem
  Holding time at the end : Subsystem
  Significant speed deviation : Subsystem
  Slope-> angle : Trigonometry  Operator=atan
  Stop Simulation : Stop
  Stop Simulation 2 : Stop
  Switch : Switch  Criteria=u2 > Threshold
  Terminator : Terminator
  altitude : Lookup_n-D  NumberOfTableDimensions=1  BreakpointsForDimension1=cycle.distance  Table=cycle.altitude  InterpMethod=Flat  RndMeth=Simplest  InputSameDT=on
  slope : Lookup_n-D  NumberOfTableDimensions=1  BreakpointsForDimension1=cycle.distance  Table=cycle.slope  InterpMethod=Flat  RndMeth=Simplest  InputSameDT=on
  speed : Lookup_n-D  NumberOfTableDimensions=1  BreakpointsForDimension1=cycle.distance  Table=cycle.speed  InterpMethod=Flat  RndMeth=Simplest  InputSameDT=on
  stop_time : Lookup_n-D  NumberOfTableDimensions=1  BreakpointsForDimension1=cycle.distance  Table=cycle.stop_time  InterpMethod=Flat  RndMeth=Simplest  InputSameDT=on
  v_target [m/s] : Outport  VectorParamsAs1DForOutWhenUnconnected=off
  stop_time [s] : Outport  Port=2  VectorParamsAs1DForOutWhenUnconnected=off
  alpha [rad] : Outport  Port=3  VectorParamsAs1DForOutWhenUnconnected=off
  tag scope: altitude=global, slope_q=global, v_target1=global
  wires:
  slope/1 -> Slope-> angle/1, [slope_q]
  Slope-> angle/1 -> alpha [rad]/1
  Compare To Constant/1 -> Holding time at the end/1(In1)
  Clock/1 -> Terminator/1  "duration"
  Switch/1 -> [v_target1], v_target [m/s]/1
  stop_time/1 -> stop_time [s]/1, Switch/2
  speed/1 -> Holding time at the beginning/1(In1)
  Holding time at the end/1(Out1) -> Stop Simulation/1
  Holding time at the beginning/1(Out1) -> Switch/3
  Constant/1 -> Switch/1
  altitude/1 -> [altitude]
  s_current [m]/1 -> slope/1, Compare To Constant/1, Significant speed deviation/1(In1), altitude/1, speed/1, stop_time/1
  Significant speed deviation/1(Out1) -> Stop Simulation 2/1

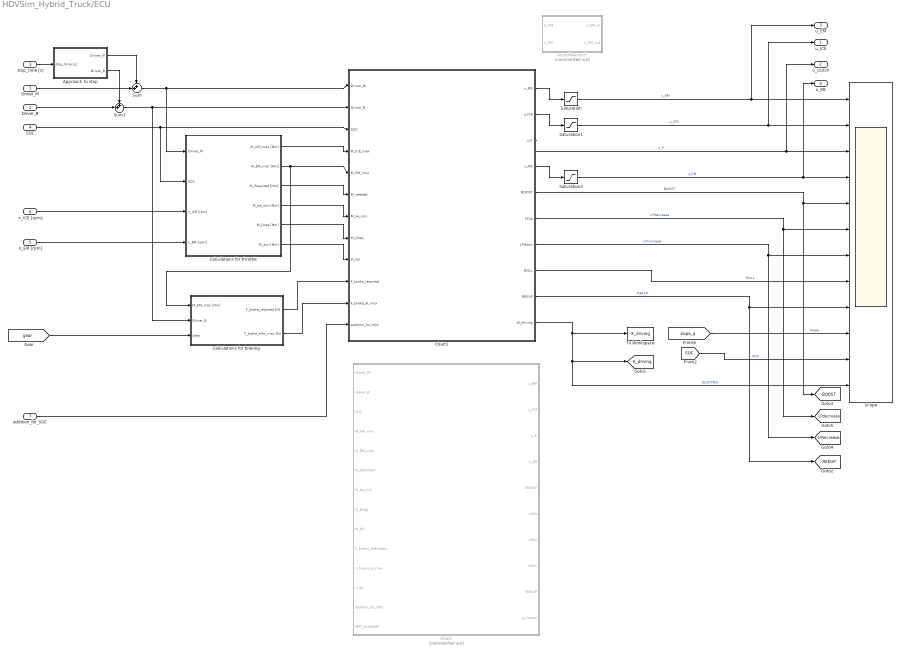
== system HDVSim_Hybrid_Truck/ECU ==
  Driver_M : Inport
  Driver_B : Inport  Port=2
  stop_time [s] : Inport  Port=3
  SOC : Inport  Port=4
  n_EM [rpm] : Inport  Port=5
  n_ICE [rpm] : Inport  Port=6
  addition_for_SOC : Inport  Port=7
  Approach to stop : Subsystem
  Ausrollversuch : Subsystem  Commented=on
  Calculations for braking : Subsystem
  Calculations for throttle : Subsystem
  Chart : Stateflow chart  Commented=on  TreatAsAtomicUnit=on
  Chart1 : Stateflow chart  TreatAsAtomicUnit=on
  Saturation : Saturate  UpperLimit=1  LowerLimit=-1
  Saturation1 : Saturate  UpperLimit=1  LowerLimit=0
  Saturation2 : Saturate  UpperLimit=1  LowerLimit=0
  Scope : Scope  NumInputPorts=12
  Sum : Sum  Inputs=++|
  Sum1 : Sum  Inputs=++|
  To Workspace : ToWorkspace  VariableName=E_driving  SampleTime=-1
      settings: Decimation=10
  u_ICE : Outport  VectorParamsAs1DForOutWhenUnconnected=off
  u_clutch : Outport  Port=2  VectorParamsAs1DForOutWhenUnconnected=off
  u_EM : Outport  Port=3  VectorParamsAs1DForOutWhenUnconnected=off
  u_KB : Outport  Port=4  VectorParamsAs1DForOutWhenUnconnected=off
  tag scope: BOOST=global, E_driving=global, LPdecrease=global, LPincrease=global, REKUP=global
  wires:
  Chart1/1(u_EM) -> Saturation/1
  Chart1/2(u_ICE) -> Saturation1/1
  Chart1/4(u_KB) -> Saturation2/1
  Sum1/1 -> Chart1/2(Driver_B), Calculations for braking/2(Driver_B)
  Sum/1 -> Chart1/1(Driver_M), Calculations for throttle/1(Driver_M)
  Chart1/3(u_K) -> u_clutch/1, Scope/3  "u_K"
  Calculations for braking/2(F_brake_elec_max [N]) -> Chart1/11(F_brake_el_max)
  Calculations for braking/1(F_brake_required [N]) -> Chart1/10(F_brake_required)
  SOC/1 -> Chart1/3(SOC), Calculations for throttle/2(SOC)
  n_ICE [rpm]/1 -> Calculations for throttle/3(n_ICE [rpm])
  Calculations for throttle/1(M_ICE_max [Nm]) -> Chart1/4(M_ICE_max)
  Calculations for throttle/3(M_Required [Nm]) -> Chart1/6(M_needed)
  Saturation1/1 -> u_ICE/1, Scope/2  "u_ICE"
  Saturation2/1 -> u_KB/1, Scope/4  "u_KB"
  Calculations for throttle/5(M_Drag [Nm]) -> Chart1/8(M_Drag)
  Calculations for throttle/4(M_be_min [Nm]) -> Chart1/7(M_be_min)
  Chart1/5(BOOST) -> [BOOST], Scope/5  "BOOST"
  Chart1/8(ROLL) -> Scope/8  "ROLL"
  Chart1/9(REKUP) -> [REKUP], Scope/9  "REKUP"
  Chart1/7(LPdown) -> [LPincrease], Scope/7  "LPincrease"
  [slope_q] -> Scope/10  "Slope"
  Calculations for throttle/6(M_aux [Nm]) -> Chart1/9(M_NV)
  Chart1/6(LPup) -> [LPdecrease], Scope/6  "LPdecrease"
  Calculations for throttle/2(M_EM_max [Nm]) -> Chart1/5(M_EM_max), Calculations for braking/1(M_EM_max [Nm])
  [SOC] -> Scope/11  "SOC"
  Saturation/1 -> u_EM/1, Scope/1  "u_EM"
  stop_time [s]/1 -> Approach to stop/1(stop_time [s])
  Approach to stop/2(Driver_B) -> Sum1/1
  Approach to stop/1(Driver_M) -> Sum/1
  Driver_M/1 -> Sum/2
  Driver_B/1 -> Sum1/2
  n_EM [rpm]/1 -> Calculations for throttle/4(n_EM [rpm])
  [gear] -> Calculations for braking/3(Gear)
  Chart1/10(el_driving) -> [E_driving], Scope/12, To Workspace/1  "ELECTRIC"
  addition_for_SOC/1 -> Chart1/12(addition_for_SOC)
